simulink model "x64_32s_001_4ks"  (Simulink 7.4)
settings: Solver=ode45  StartTime=0.0  StopTime=100000  RelTol=1e-3
undefined symbols (not defined in the file): gcbh, ROACH, x64_32s_001_4ks_beam_mux_order_in_user_data_out, x64_32s_001_4ks_beam_mux_order_out_user_data_out, x64_32s_001_4ks_beam_mux_order_we_user_data_out, x64_32s_001_4ks_first_chan_user_data_out, x64_32s_001_4ks_gbe_dest_ip_user_data_out, x64_32s_001_4ks_gbe_dest_port_user_data_out, x64_32s_001_4ks_gbe_packet_cnt1_user_data_in, x64_32s_001_4ks_gbe_packet_cnt_user_data_in, x64_32s_001_4ks_heap_size_user_data_out, x64_32s_001_4ks_inst_seng_id0_user_data_in, x64_32s_001_4ks_last_chan_user_data_out, x64_32s_001_4ks_n_beams_user_data_out, x64_32s_001_4ks_qdr_in_cnt_user_data_in, x64_32s_001_4ks_status_user_data_in, x64_32s_001_4ks_x_window_mult_0_coeff_user_data_out, x64_32s_001_4ks_x_window_mult_1_coeff_user_data_out, x64_32s_001_4ks_x_window_mult_2_coeff_user_data_out, x64_32s_001_4ks_x_window_mult_3_coeff_user_data_out, x64_32s_001_4ks_xaui_cnt0_user_data_in, x64_32s_001_4ks_xaui_error_cnt0_user_data_in, x64_32s_001_4ks_xauibuf_out_cnt_user_data_in, x64_32s_001_4ks_xauibuf_window_cnt_user_data_in, x64_32s_001_4ks_y_window_mult_0_coeff_user_data_out, x64_32s_001_4ks_y_window_mult_1_coeff_user_data_out, x64_32s_001_4ks_y_window_mult_2_coeff_user_data_out, x64_32s_001_4ks_y_window_mult_3_coeff_user_data_out, x64_32s_001_4ks_y_window_mult_4_coeff_user_data_out, x64_32s_001_4ks_y_window_mult_5_coeff_user_data_out, x64_32s_001_4ks_y_window_mult_6_coeff_user_data_out, x64_32s_001_4ks_y_window_mult_7_coeff_user_data_out, xlBlockLoadCallback
libraries: xbsIndex_r4 x8559, casper_library_misc x308, casper_library_delays x33, casper_library_reorder x16, xps_library x5, ox_lib x1
(part 3 of 100)

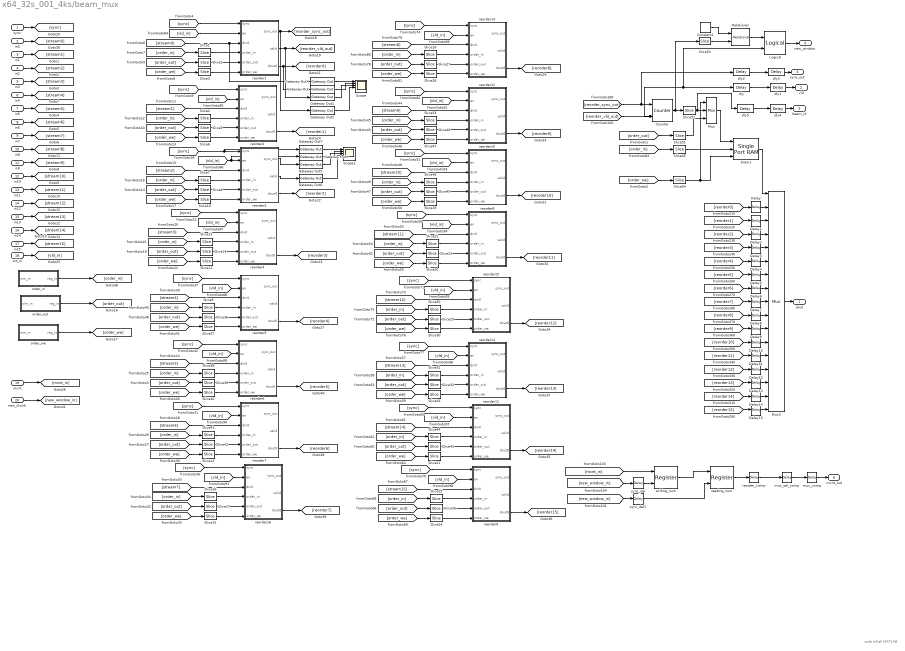
== system x64_32s_001_4ks/beam_mux ==
  note: sudo killall MATLAB
  sync : Inport
  in0 : Inport  Port=2
  in1 : Inport  Port=3
  in2 : Inport  Port=4
  in3 : Inport  Port=5
  in4 : Inport  Port=6
  in5 : Inport  Port=7
  in6 : Inport  Port=8
  in7 : Inport  Port=9
  in8 : Inport  Port=10
  in9 : Inport  Port=11
  in10 : Inport  Port=12
  in11 : Inport  Port=13
  in12 : Inport  Port=14
  in13 : Inport  Port=15
  in14 : Inport  Port=16
  in15 : Inport  Port=17
  vld_in : Inport  Port=18
  mcnt : Inport  Port=19
  new_chunk : Inport  Port=20
  Constant1 : xbsIndex_r4/Constant  arith_type=Unsigned  const=0  n_bits=n_int  bin_pt=0  explicit_period=on  period=1  equ=P=C  opselect=C  inp2=PCIN>>17  opr=+  inp1=P  carry=CIN  dbl_ovrd=off  block_type=constant
      settings: block_version=9.2.01
  Counter : xbsIndex_r4/Counter  cnt_type=Count Limited  cnt_to=(2^(n_int+n_mux))-1  operation=Up  start_count=0  cnt_by_val=1  arith_type=Unsigned  n_bits=n_int+n_mux  bin_pt=0  load_pin=off  rst=on  en=on  explicit_period=on  period=1  dbl_ovrd=off  use_behavioral_HDL=off  implementation=Fabric  block_type=counter
      settings: block_version=11.4
  Delay{,1..15} (16 alike) : xbsIndex_r4/Delay  en=off  latency=1  dbl_ovrd=off  reg_retiming=on  block_type=delay
      settings: xl_area=[0, 0, 0, 0, 0, 0, 0]  block_version=9.2.01
  Gateway Out{,1..7} (8 alike) : xbsIndex_r4/Gateway Out  hdl_port=off  timing_constraint=None  locs_specified=off  LOCs={}  block_type=gatewayout
      settings: infoedit="Gateway out block.  Converts Xilinx fixed point inputs into ouputs of type Simulink integer, double, or fixed point.<br><br>Hardware notes:  In hardware these blocks become top level output ports or are discarded, depending on how they are configured."  block_version=11.4
  Logical : xbsIndex_r4/Logical  logical_function=AND  inputs=2  en=off  latency=2  precision=Full  arith_type=Unsigned  n_bits=16  bin_pt=0  align_bp=on  dbl_ovrd=off  block_type=logical
      settings: block_version=11.4
  Mux : xbsIndex_r4/Mux  inputs=2  en=off  latency=0  precision=Full  arith_type="Signed  (2's comp)"  n_bits=8  bin_pt=2  quantization=Truncate  overflow=Wrap  dbl_ovrd=off  block_type=mux
      settings: xl_area=[0, 0, 0, 0, 0, 0, 0]  block_version=9.1.01
  Mux3 : xbsIndex_r4/Mux  inputs=16  en=off  latency=2  precision=Full  arith_type="Signed  (2's comp)"  n_bits=0  bin_pt=0  quantization=Truncate  overflow=Wrap  dbl_ovrd=off  block_type=mux
      settings: xl_area=[0, 0, 0, 0, 0, 0, 0]  block_version=9.2.01
  Relational : xbsIndex_r4/Relational  mode=a=b  en=off  latency=0  dbl_ovrd=off  block_type=relational
      settings: xl_area=[0, 0, 0, 0, 0, 0, 0]  block_version=9.2.01
  Scope : Scope  NumInputPorts=6
      settings: YMin=-5~-5~-5~-5~-5~-5  YMax=5~5~5~5~5~5
  Scope1 : Scope  NumInputPorts=6
      settings: YMin=-5~-5~-5~-5~-5~-5  YMax=5~5~5~5~5~5  SaveName=ScopeData7
  Slice{1..2,4..5,7..9,13..14,16..18,22..23,25..27,29,31..33,36,38..39,41..42,44..46,48..51} (33 alike) : xbsIndex_r4/Slice  nbits=n_mux  boolean_output=off  mode=Lower Bit Location + Width  bit1=0  base1=MSB of Input  bit0=0  base0=LSB of Input  dbl_ovrd=off  block_type=slice
  Slice10 : xbsIndex_r4/Slice  nbits=1  boolean_output=on  mode=Lower Bit Location + Width  bit1=0  base1=MSB of Input  bit0=2  base0=LSB of Input  dbl_ovrd=off  block_type=slice
  Slice11 : xbsIndex_r4/Slice  nbits=1  boolean_output=on  mode=Lower Bit Location + Width  bit1=0  base1=MSB of Input  bit0=3  base0=LSB of Input  dbl_ovrd=off  block_type=slice
  Slice12 : xbsIndex_r4/Slice  nbits=1  boolean_output=on  mode=Lower Bit Location + Width  bit1=0  base1=MSB of Input  bit0=6  base0=LSB of Input  dbl_ovrd=off  block_type=slice
  Slice15 : xbsIndex_r4/Slice  nbits=1  boolean_output=on  mode=Lower Bit Location + Width  bit1=0  base1=MSB of Input  bit0=7  base0=LSB of Input  dbl_ovrd=off  block_type=slice
  Slice19 : xbsIndex_r4/Slice  nbits=1  boolean_output=on  mode=Lower Bit Location + Width  bit1=0  base1=MSB of Input  bit0=10  base0=LSB of Input  dbl_ovrd=off  block_type=slice
  Slice20 : xbsIndex_r4/Slice  nbits=1  boolean_output=on  mode=Lower Bit Location + Width  bit1=0  base1=MSB of Input  bit0=11  base0=LSB of Input  dbl_ovrd=off  block_type=slice
  Slice21 : xbsIndex_r4/Slice  nbits=1  boolean_output=on  mode=Lower Bit Location + Width  bit1=0  base1=MSB of Input  bit0=14  base0=LSB of Input  dbl_ovrd=off  block_type=slice
  Slice24 : xbsIndex_r4/Slice  nbits=1  boolean_output=on  mode=Lower Bit Location + Width  bit1=0  base1=MSB of Input  bit0=15  base0=LSB of Input  dbl_ovrd=off  block_type=slice
  Slice28 : xbsIndex_r4/Slice  nbits=1  boolean_output=on  mode=Lower Bit Location + Width  bit1=0  base1=MSB of Input  bit0=8  base0=LSB of Input  dbl_ovrd=off  block_type=slice
  Slice3 : xbsIndex_r4/Slice  nbits=1  boolean_output=on  mode=Lower Bit Location + Width  bit1=0  base1=MSB of Input  bit0=0  base0=LSB of Input  dbl_ovrd=off  block_type=slice
  Slice30 : xbsIndex_r4/Slice  nbits=1  boolean_output=on  mode=Lower Bit Location + Width  bit1=0  base1=MSB of Input  bit0=12  base0=LSB of Input  dbl_ovrd=off  block_type=slice
  Slice34 : xbsIndex_r4/Slice  nbits=1  boolean_output=on  mode=Upper Bit Location + Width  bit1=0  base1=MSB of Input  bit0=11  base0=LSB of Input  dbl_ovrd=off  block_type=slice
  Slice35 : xbsIndex_r4/Slice  nbits=log2(16)  boolean_output=off  mode=Lower Bit Location + Width  bit1=0  base1=MSB of Input  bit0=0  base0=LSB of Input  dbl_ovrd=off  block_type=slice
  Slice37 : xbsIndex_r4/Slice  nbits=1  boolean_output=on  mode=Lower Bit Location + Width  bit1=0  base1=MSB of Input  bit0=4  base0=LSB of Input  dbl_ovrd=off  block_type=slice
  Slice40 : xbsIndex_r4/Slice  nbits=1  boolean_output=on  mode=Lower Bit Location + Width  bit1=0  base1=MSB of Input  bit0=5  base0=LSB of Input  dbl_ovrd=off  block_type=slice
  Slice43 : xbsIndex_r4/Slice  nbits=1  boolean_output=on  mode=Lower Bit Location + Width  bit1=0  base1=MSB of Input  bit0=13  base0=LSB of Input  dbl_ovrd=off  block_type=slice
  Slice47 : xbsIndex_r4/Slice  nbits=1  boolean_output=on  mode=Lower Bit Location + Width  bit1=0  base1=MSB of Input  bit0=9  base0=LSB of Input  dbl_ovrd=off  block_type=slice
  Slice52 : xbsIndex_r4/Slice  nbits=n_mux  boolean_output=off  mode=Upper Bit Location + Width  bit1=0  base1=MSB of Input  bit0=0  base0=LSB of Input  dbl_ovrd=off  block_type=slice
  Slice53 : xbsIndex_r4/Slice  nbits=n_int  boolean_output=off  mode=Lower Bit Location + Width  bit1=0  base1=MSB of Input  bit0=0  base0=LSB of Input  dbl_ovrd=off  block_type=slice
  Slice6 : xbsIndex_r4/Slice  nbits=1  boolean_output=on  mode=Lower Bit Location + Width  bit1=0  base1=MSB of Input  bit0=1  base0=LSB of Input  dbl_ovrd=off  block_type=slice
  bram1 : xbsIndex_r4/Single Port RAM  depth=2^n_mux  initVector=[0 0 0 0 1 1 1 1 0 0 0 0 1 1 1 1]  distributed_mem=Distributed memory  write_mode=Read Before Write  rst=off  init_reg=0  en=off  latency=1  dbl_ovrd=off  optimize=Area  use_rpm=off  block_type=spram
      settings: xl_area=[0, 0, 0, 0, 0, 0, 0]  block_version=9.1.01
  dly : xbsIndex_r4/Delay  en=off  latency=1  dbl_ovrd=off  reg_retiming=off  block_type=delay
      settings: block_version=11.4
  dly1 : xbsIndex_r4/Delay  en=off  latency=2  dbl_ovrd=off  reg_retiming=off  block_type=delay
      settings: block_version=11.4
  dly2 : xbsIndex_r4/Delay  en=off  latency=1  dbl_ovrd=off  reg_retiming=off  block_type=delay
      settings: block_version=11.4
  dly3 : xbsIndex_r4/Delay  en=off  latency=2  dbl_ovrd=off  reg_retiming=off  block_type=delay
      settings: block_version=11.4
  dly4 : xbsIndex_r4/Delay  en=off  latency=2  dbl_ovrd=off  reg_retiming=off  block_type=delay
      settings: block_version=11.4
  dly5 : xbsIndex_r4/Delay  en=off  latency=1  dbl_ovrd=off  reg_retiming=off  block_type=delay
      settings: block_version=11.4
  mux_comp : xbsIndex_r4/Delay  en=off  latency=2  dbl_ovrd=off  reg_retiming=on  block_type=delay
      settings: xl_area=[0, 0, 0, 0, 0, 0, 0]  block_version=9.2.01
  mux_sel_comp : xbsIndex_r4/Delay  en=off  latency=1  dbl_ovrd=off  reg_retiming=on  block_type=delay
      settings: xl_area=[0, 0, 0, 0, 0, 0, 0]  block_version=9.2.01
  order_in : Subsystem  Tag=xps:sw_reg  mask{io_dir=From Processor, arith_type=Unsigned, bitwidth=32, bin_pt=0, sample_period=1, gw_name=x64_32s_001_4ks_beam_mux_order_in_user_data_out}
      mask prompts: io_dir "I/O direction"; arith_type "Data Type"; bitwidth "Data bitwidth"; bin_pt "Data binary point"; sample_period "Sample period"; gw_name "Mask Parameter 6:"
      mask init:
      | register_mask;
      mask StyleString: popup(From Processor|To Processor),popup(Unsigned|Signed  (2's comp)),edit,edit,edit,edit
  order_out : Subsystem  Tag=xps:sw_reg  mask{io_dir=From Processor, arith_type=Unsigned, bitwidth=32, bin_pt=0, sample_period=1, gw_name=x64_32s_001_4ks_beam_mux_order_out_user_data_out}
      mask prompts: io_dir "I/O direction"; arith_type "Data Type"; bitwidth "Data bitwidth"; bin_pt "Data binary point"; sample_period "Sample period"; gw_name "Mask Parameter 6:"
      mask init:
      | register_mask;
      mask StyleString: popup(From Processor|To Processor),popup(Unsigned|Signed  (2's comp)),edit,edit,edit,edit
  order_we : Subsystem  Tag=xps:sw_reg  mask{io_dir=From Processor, arith_type=Unsigned, bitwidth=32, bin_pt=0, sample_period=1, gw_name=x64_32s_001_4ks_beam_mux_order_we_user_data_out}
      mask prompts: io_dir "I/O direction"; arith_type "Data Type"; bitwidth "Data bitwidth"; bin_pt "Data binary point"; sample_period "Sample period"; gw_name "Mask Parameter 6:"
      mask init:
      | register_mask;
      mask StyleString: popup(From Processor|To Processor),popup(Unsigned|Signed  (2's comp)),edit,edit,edit,edit
  reading_num : xbsIndex_r4/Register  init=0  rst=off  en=on  dbl_ovrd=off  block_type=register
      settings: block_version=11.4
  reorder1 : Subsystem  AncestorBlock=casper_library_reorder/reorder  mask(reorder){map=getfield( getfield( get_param( gcb,'UserData' ), 'parameters'),'map'), n_inputs=1, bram_latency=1, map_latency=1, double_buffer=1, bram_map=off}
      mask prompts: map "Output Order:"; n_inputs "Number of inputs"; bram_latency "BRAM Latency"; map_latency "Map Latency"; double_buffer "Double Buffer"; bram_map "Use BlockRAM for Map"
      settings: AttributesFormatString=order=2
      mask Help: eval('xlWeb(''http://casper.berkeley.edu/wiki/Reorder'')')
  reorder10 : Subsystem  AncestorBlock=casper_library_reorder/reorder  mask(reorder){map=getfield( getfield( get_param( gcb,'UserData' ), 'parameters'),'map'), n_inputs=1, bram_latency=1, map_latency=1, double_buffer=1, bram_map=off}
      mask prompts: map "Output Order:"; n_inputs "Number of inputs"; bram_latency "BRAM Latency"; map_latency "Map Latency"; double_buffer "Double Buffer"; bram_map "Use BlockRAM for Map"
      settings: AttributesFormatString=order=2
      mask Help: eval('xlWeb(''http://casper.berkeley.edu/wiki/Reorder'')')
  reorder11 : Subsystem  AncestorBlock=casper_library_reorder/reorder  mask(reorder){map=getfield( getfield( get_param( gcb,'UserData' ), 'parameters'),'map'), n_inputs=1, bram_latency=1, map_latency=1, double_buffer=1, bram_map=off}
      mask prompts: map "Output Order:"; n_inputs "Number of inputs"; bram_latency "BRAM Latency"; map_latency "Map Latency"; double_buffer "Double Buffer"; bram_map "Use BlockRAM for Map"
      settings: AttributesFormatString=order=2
      mask Help: eval('xlWeb(''http://casper.berkeley.edu/wiki/Reorder'')')
  reorder12 : Subsystem  AncestorBlock=casper_library_reorder/reorder  mask(reorder){map=getfield( getfield( get_param( gcb,'UserData' ), 'parameters'),'map'), n_inputs=1, bram_latency=1, map_latency=1, double_buffer=1, bram_map=off}
      mask prompts: map "Output Order:"; n_inputs "Number of inputs"; bram_latency "BRAM Latency"; map_latency "Map Latency"; double_buffer "Double Buffer"; bram_map "Use BlockRAM for Map"
      settings: AttributesFormatString=order=2
      mask Help: eval('xlWeb(''http://casper.berkeley.edu/wiki/Reorder'')')
  reorder13 : Subsystem  AncestorBlock=casper_library_reorder/reorder  mask(reorder){map=getfield( getfield( get_param( gcb,'UserData' ), 'parameters'),'map'), n_inputs=1, bram_latency=1, map_latency=1, double_buffer=1, bram_map=off}
      mask prompts: map "Output Order:"; n_inputs "Number of inputs"; bram_latency "BRAM Latency"; map_latency "Map Latency"; double_buffer "Double Buffer"; bram_map "Use BlockRAM for Map"
      settings: AttributesFormatString=order=2
      mask Help: eval('xlWeb(''http://casper.berkeley.edu/wiki/Reorder'')')
  reorder14 : Subsystem  AncestorBlock=casper_library_reorder/reorder  mask(reorder){map=getfield( getfield( get_param( gcb,'UserData' ), 'parameters'),'map'), n_inputs=1, bram_latency=1, map_latency=1, double_buffer=1, bram_map=off}
      mask prompts: map "Output Order:"; n_inputs "Number of inputs"; bram_latency "BRAM Latency"; map_latency "Map Latency"; double_buffer "Double Buffer"; bram_map "Use BlockRAM for Map"
      settings: AttributesFormatString=order=2
      mask Help: eval('xlWeb(''http://casper.berkeley.edu/wiki/Reorder'')')
  reorder15 : Subsystem  AncestorBlock=casper_library_reorder/reorder  mask(reorder){map=getfield( getfield( get_param( gcb,'UserData' ), 'parameters'),'map'), n_inputs=1, bram_latency=1, map_latency=1, double_buffer=1, bram_map=off}
      mask prompts: map "Output Order:"; n_inputs "Number of inputs"; bram_latency "BRAM Latency"; map_latency "Map Latency"; double_buffer "Double Buffer"; bram_map "Use BlockRAM for Map"
      settings: AttributesFormatString=order=2
      mask Help: eval('xlWeb(''http://casper.berkeley.edu/wiki/Reorder'')')
  reorder16 : Subsystem  AncestorBlock=casper_library_reorder/reorder  mask(reorder){map=getfield( getfield( get_param( gcb,'UserData' ), 'parameters'),'map'), n_inputs=1, bram_latency=1, map_latency=1, double_buffer=1, bram_map=off}
      mask prompts: map "Output Order:"; n_inputs "Number of inputs"; bram_latency "BRAM Latency"; map_latency "Map Latency"; double_buffer "Double Buffer"; bram_map "Use BlockRAM for Map"
      settings: AttributesFormatString=order=2
      mask Help: eval('xlWeb(''http://casper.berkeley.edu/wiki/Reorder'')')
  reorder2 : Subsystem  AncestorBlock=casper_library_reorder/reorder  mask(reorder){map=getfield( getfield( get_param( gcb,'UserData' ), 'parameters'),'map'), n_inputs=1, bram_latency=1, map_latency=1, double_buffer=1, bram_map=off}
      mask prompts: map "Output Order:"; n_inputs "Number of inputs"; bram_latency "BRAM Latency"; map_latency "Map Latency"; double_buffer "Double Buffer"; bram_map "Use BlockRAM for Map"
      settings: AttributesFormatString=order=2
      mask Help: eval('xlWeb(''http://casper.berkeley.edu/wiki/Reorder'')')
  reorder3 : Subsystem  AncestorBlock=casper_library_reorder/reorder  mask(reorder){map=getfield( getfield( get_param( gcb,'UserData' ), 'parameters'),'map'), n_inputs=1, bram_latency=1, map_latency=1, double_buffer=1, bram_map=off}
      mask prompts: map "Output Order:"; n_inputs "Number of inputs"; bram_latency "BRAM Latency"; map_latency "Map Latency"; double_buffer "Double Buffer"; bram_map "Use BlockRAM for Map"
      settings: AttributesFormatString=order=2
      mask Help: eval('xlWeb(''http://casper.berkeley.edu/wiki/Reorder'')')
  reorder4 : Subsystem  AncestorBlock=casper_library_reorder/reorder  mask(reorder){map=getfield( getfield( get_param( gcb,'UserData' ), 'parameters'),'map'), n_inputs=1, bram_latency=1, map_latency=1, double_buffer=1, bram_map=off}
      mask prompts: map "Output Order:"; n_inputs "Number of inputs"; bram_latency "BRAM Latency"; map_latency "Map Latency"; double_buffer "Double Buffer"; bram_map "Use BlockRAM for Map"
      settings: AttributesFormatString=order=2
      mask Help: eval('xlWeb(''http://casper.berkeley.edu/wiki/Reorder'')')
  reorder5 : Subsystem  AncestorBlock=casper_library_reorder/reorder  mask(reorder){map=getfield( getfield( get_param( gcb,'UserData' ), 'parameters'),'map'), n_inputs=1, bram_latency=1, map_latency=1, double_buffer=1, bram_map=off}
      mask prompts: map "Output Order:"; n_inputs "Number of inputs"; bram_latency "BRAM Latency"; map_latency "Map Latency"; double_buffer "Double Buffer"; bram_map "Use BlockRAM for Map"
      settings: AttributesFormatString=order=2
      mask Help: eval('xlWeb(''http://casper.berkeley.edu/wiki/Reorder'')')
  reorder6 : Subsystem  AncestorBlock=casper_library_reorder/reorder  mask(reorder){map=getfield( getfield( get_param( gcb,'UserData' ), 'parameters'),'map'), n_inputs=1, bram_latency=1, map_latency=1, double_buffer=1, bram_map=off}
      mask prompts: map "Output Order:"; n_inputs "Number of inputs"; bram_latency "BRAM Latency"; map_latency "Map Latency"; double_buffer "Double Buffer"; bram_map "Use BlockRAM for Map"
      settings: AttributesFormatString=order=2
      mask Help: eval('xlWeb(''http://casper.berkeley.edu/wiki/Reorder'')')
  reorder7 : Subsystem  AncestorBlock=casper_library_reorder/reorder  mask(reorder){map=getfield( getfield( get_param( gcb,'UserData' ), 'parameters'),'map'), n_inputs=1, bram_latency=1, map_latency=1, double_buffer=1, bram_map=off}
      mask prompts: map "Output Order:"; n_inputs "Number of inputs"; bram_latency "BRAM Latency"; map_latency "Map Latency"; double_buffer "Double Buffer"; bram_map "Use BlockRAM for Map"
      settings: AttributesFormatString=order=2
      mask Help: eval('xlWeb(''http://casper.berkeley.edu/wiki/Reorder'')')
  reorder8 : Subsystem  AncestorBlock=casper_library_reorder/reorder  mask(reorder){map=getfield( getfield( get_param( gcb,'UserData' ), 'parameters'),'map'), n_inputs=1, bram_latency=1, map_latency=1, double_buffer=1, bram_map=off}
      mask prompts: map "Output Order:"; n_inputs "Number of inputs"; bram_latency "BRAM Latency"; map_latency "Map Latency"; double_buffer "Double Buffer"; bram_map "Use BlockRAM for Map"
      settings: AttributesFormatString=order=2
      mask Help: eval('xlWeb(''http://casper.berkeley.edu/wiki/Reorder'')')
  reorder9 : Subsystem  AncestorBlock=casper_library_reorder/reorder  mask(reorder){map=getfield( getfield( get_param( gcb,'UserData' ), 'parameters'),'map'), n_inputs=1, bram_latency=1, map_latency=1, double_buffer=1, bram_map=off}
      mask prompts: map "Output Order:"; n_inputs "Number of inputs"; bram_latency "BRAM Latency"; map_latency "Map Latency"; double_buffer "Double Buffer"; bram_map "Use BlockRAM for Map"
      settings: AttributesFormatString=order=2
      mask Help: eval('xlWeb(''http://casper.berkeley.edu/wiki/Reorder'')')
  reorder_comp : xbsIndex_r4/Delay  en=off  latency=3  dbl_ovrd=off  reg_retiming=on  block_type=delay
      settings: xl_area=[0, 0, 0, 0, 0, 0, 0]  block_version=9.2.01
  sync_del : xbsIndex_r4/Delay  en=off  latency=1  dbl_ovrd=off  reg_retiming=on  block_type=delay
      settings: xl_area=[0, 0, 0, 0, 0, 0, 0]  block_version=9.2.01
  sync_del1 : xbsIndex_r4/Delay  en=off  latency=1  dbl_ovrd=off  reg_retiming=on  block_type=delay
      settings: xl_area=[0, 0, 0, 0, 0, 0, 0]  block_version=9.2.01
  writing_num : xbsIndex_r4/Register  init=0  rst=off  en=on  dbl_ovrd=off  block_type=register
      settings: block_version=11.4
  dout : Outport
  vld : Outport  Port=2
  sync_out : Outport  Port=3
  new_window : Outport  Port=4
  beam_id : Outport  Port=5
  mcnt_out : Outport  Port=6
  wires:
  in0/1 -> [stream0]
  in1/1 -> [stream1]
  in2/1 -> [stream2]
  in3/1 -> [stream3]
  in4/1 -> [stream4]
  in5/1 -> [stream5]
  in6/1 -> [stream6]
  in7/1 -> [stream7]
  in8/1 -> [stream8]
  in9/1 -> [stream9]
  in10/1 -> [stream10]
  in11/1 -> [stream11]
  in12/1 -> [stream12]
  in13/1 -> [stream13]
  in14/1 -> [stream14]
  in15/1 -> [stream15]
  [reorder0] -> Delay/1
  [reorder1] -> Delay1/1
  [reorder2] -> Delay2/1
  [reorder3] -> Delay3/1
  [reorder4] -> Delay4/1
  [reorder5] -> Delay5/1
  [reorder6] -> Delay6/1
  [reorder7] -> Delay7/1
  [reorder8] -> Delay8/1
  [reorder9] -> Delay9/1
  [reorder10] -> Delay10/1
  [reorder11] -> Delay11/1
  [reorder12] -> Delay12/1
  [reorder13] -> Delay13/1
  [reorder14] -> Delay14/1
  [reorder15] -> Delay15/1
  Mux3/1 -> dout/1
  sync/1 -> [sync]
  order_out/1(reg_in) -> [order_out]
  order_we/1(reg_in) -> [order_we]
  reorder1/1(sync_out) -> [reorder_sync_out], Gateway Out2/1
  reorder1/2(valid) -> [reorder_vld_out], Gateway Out1/1
  [reorder_sync_out] -> Counter/1, dly2/1
  [reorder_vld_out] -> Counter/2, dly/1, Logical/2
  [order_we] -> Slice34/1
  Slice34/1 -> bram1/3, Mux/1
  Slice35/1 -> bram1/2
  order_in/1(reg_in) -> [order_in]
  [sync] -> reorder1/1(sync)
  [vld_in] -> reorder1/2(en)
  [stream0] -> Gateway Out4/1, reorder1/3(din0)
  [order_in] -> Slice1/1
  [order_we] -> Slice3/1
  Slice1/1 -> reorder1/4(order_in)
  Slice3/1 -> reorder1/6(order_we)
  reorder1/3(dout0) -> [reorder0], Gateway Out/1
  [order_out] -> Slice2/1
  Slice2/1 -> reorder1/5(order_out)
  [sync] -> reorder2/1(sync)
  [vld_in] -> reorder2/2(en)
  [stream1] -> reorder2/3(din0)
  [order_in] -> Slice4/1
  [order_we] -> Slice6/1
  Slice4/1 -> reorder2/4(order_in)
  Slice6/1 -> reorder2/6(order_we)
  reorder2/3(dout0) -> [reorder1]
  [order_out] -> Slice5/1
  Slice5/1 -> reorder2/5(order_out)
  [sync] -> Gateway Out7/1, reorder3/1(sync)
  [vld_in] -> Gateway Out3/1, reorder3/2(en)
  [stream2] -> reorder3/3(din0)
  [order_in] -> Slice7/1
  [order_we] -> Slice10/1
  Slice7/1 -> reorder3/4(order_in)
  Slice10/1 -> reorder3/6(order_we)
  reorder3/3(dout0) -> [reorder2]
  [order_out] -> Slice8/1
  Slice8/1 -> reorder3/5(order_out)
  [sync] -> reorder4/1(sync)
  [vld_in] -> reorder4/2(en)
  [stream3] -> reorder4/3(din0)
  [order_in] -> Slice13/1
  [order_we] -> Slice11/1
  Slice13/1 -> reorder4/4(order_in)
  Slice11/1 -> reorder4/6(order_we)
  reorder4/3(dout0) -> [reorder3]
  [order_out] -> Slice14/1
  Slice14/1 -> reorder4/5(order_out)
  [sync] -> reorder5/1(sync)
  [vld_in] -> reorder5/2(en)
  [stream4] -> reorder5/3(din0)
  [order_in] -> Slice9/1
  [order_we] -> Slice37/1
  Slice9/1 -> reorder5/4(order_in)
  Slice37/1 -> reorder5/6(order_we)
  reorder5/3(dout0) -> [reorder4]
  [order_out] -> Slice36/1
  Slice36/1 -> reorder5/5(order_out)
  [sync] -> reorder6/1(sync)
  [vld_in] -> reorder6/2(en)
  [stream5] -> reorder6/3(din0)
  [order_in] -> Slice38/1
  [order_we] -> Slice40/1
  Slice38/1 -> reorder6/4(order_in)
  Slice40/1 -> reorder6/6(order_we)
  reorder6/3(dout0) -> [reorder5]
  [order_out] -> Slice39/1
  Slice39/1 -> reorder6/5(order_out)
  [sync] -> reorder7/1(sync)
  [vld_in] -> reorder7/2(en)
  [stream6] -> reorder7/3(din0)
  [order_in] -> Slice41/1
  [order_we] -> Slice12/1
  Slice41/1 -> reorder7/4(order_in)
  Slice12/1 -> reorder7/6(order_we)
  reorder7/3(dout0) -> [reorder6]
  [order_out] -> Slice42/1
  Slice42/1 -> reorder7/5(order_out)
  [sync] -> reorder16/1(sync)
  [vld_in] -> reorder16/2(en)
  [stream7] -> reorder16/3(din0)
  [order_in] -> Slice16/1
  [order_we] -> Slice15/1
  Slice16/1 -> reorder16/4(order_in)
  Slice15/1 -> reorder16/6(order_we)
  reorder16/3(dout0) -> [reorder7]
  [order_out] -> Slice17/1
  Slice17/1 -> reorder16/5(order_out)
  [sync] -> reorder13/1(sync)
  [vld_in] -> reorder13/2(en)
  [stream8] -> reorder13/3(din0)
  [order_in] -> Slice18/1
  [order_we] -> Slice28/1
  Slice18/1 -> reorder13/4(order_in)
  Slice28/1 -> reorder13/6(order_we)
  reorder13/3(dout0) -> [reorder8]
  [order_out] -> Slice27/1
  Slice27/1 -> reorder13/5(order_out)
  [sync] -> reorder12/1(sync)
  [vld_in] -> reorder12/2(en)
  [stream9] -> reorder12/3(din0)
  [order_in] -> Slice33/1
  [order_we] -> Slice47/1
  Slice33/1 -> reorder12/4(order_in)
  Slice47/1 -> reorder12/6(order_we)
  reorder12/3(dout0) -> [reorder9]
  [order_out] -> Slice46/1
  Slice46/1 -> reorder12/5(order_out)
  [sync] -> reorder10/1(sync)
  [vld_in] -> reorder10/2(en)
  [stream10] -> reorder10/3(din0)
  [order_in] -> Slice48/1
  [order_we] -> Slice19/1
  Slice48/1 -> reorder10/4(order_in)
  Slice19/1 -> reorder10/6(order_we)
  reorder10/3(dout0) -> [reorder10]
  [order_out] -> Slice49/1
  Slice49/1 -> reorder10/5(order_out)
  [sync] -> reorder8/1(sync)
  [vld_in] -> reorder8/2(en)
  [stream11] -> reorder8/3(din0)
  [order_in] -> Slice22/1
  [order_we] -> Slice20/1
  Slice22/1 -> reorder8/4(order_in)
  Slice20/1 -> reorder8/6(order_we)
  reorder8/3(dout0) -> [reorder11]
  [order_out] -> Slice23/1
  Slice23/1 -> reorder8/5(order_out)
  [sync] -> reorder15/1(sync)
  [vld_in] -> reorder15/2(en)
  [stream12] -> reorder15/3(din0)
  [order_in] -> Slice50/1
  [order_we] -> Slice30/1
  Slice50/1 -> reorder15/4(order_in)
  Slice30/1 -> reorder15/6(order_we)
  reorder15/3(dout0) -> [reorder12]
  [order_out] -> Slice29/1
  Slice29/1 -> reorder15/5(order_out)
  [sync] -> reorder14/1(sync)
  [vld_in] -> reorder14/2(en)
  [stream13] -> reorder14/3(din0)
  [order_in] -> Slice31/1
  [order_we] -> Slice43/1
  Slice31/1 -> reorder14/4(order_in)
  Slice43/1 -> reorder14/6(order_we)
  reorder14/3(dout0) -> [reorder13]
  [order_out] -> Slice32/1
  Slice32/1 -> reorder14/5(order_out)
  [sync] -> reorder11/1(sync)
  [vld_in] -> reorder11/2(en)
  [stream14] -> reorder11/3(din0)
  [order_in] -> Slice44/1
  [order_we] -> Slice21/1
  Slice44/1 -> reorder11/4(order_in)
  Slice21/1 -> reorder11/6(order_we)
  reorder11/3(dout0) -> [reorder14]
  [order_out] -> Slice45/1
  Slice45/1 -> reorder11/5(order_out)
  [sync] -> reorder9/1(sync)
  [vld_in] -> reorder9/2(en)
  [stream15] -> reorder9/3(din0)
  [order_in] -> Slice25/1
  [order_we] -> Slice24/1
  Slice25/1 -> reorder9/4(order_in)
  Slice24/1 -> reorder9/6(order_we)
  reorder9/3(dout0) -> [reorder15]
  [order_out] -> Slice26/1
  Slice26/1 -> reorder9/5(order_out)
  Counter/1 -> Slice52/1, Slice53/1
  Slice51/1 -> Mux/3
  Mux/1 -> bram1/1
  dly/1 -> dly1/1
  dly1/1 -> vld/1
  Slice52/1 -> Mux/2, dly5/1
  dly2/1 -> dly3/1
  dly3/1 -> sync_out/1
  vld_in/1 -> [vld_in]
  Gateway Out4/1 -> Scope/1
  Gateway Out/1 -> Scope/2
  Gateway Out1/1 -> Scope/3
  Delay/1 -> Mux3/2
  Delay1/1 -> Mux3/3
  Delay2/1 -> Mux3/4
  Delay3/1 -> Mux3/5
  Delay4/1 -> Mux3/6
  Delay5/1 -> Mux3/7
  Delay6/1 -> Mux3/8
  Delay7/1 -> Mux3/9
  Delay8/1 -> Mux3/10
  Delay9/1 -> Mux3/11
  Delay10/1 -> Mux3/12
  Delay11/1 -> Mux3/13
  Delay12/1 -> Mux3/14
  Delay13/1 -> Mux3/15
  Delay14/1 -> Mux3/16
  Delay15/1 -> Mux3/17
  Constant1/1 -> Relational/1
  Relational/1 -> Logical/1
  Logical/1 -> new_window/1
  Slice53/1 -> Relational/2
  dly4/1 -> beam_id/1
  bram1/1 -> Mux3/1
  dly5/1 -> dly4/1
  writing_num/1 -> reading_num/1
  mcnt/1 -> [mcnt_in]
  [mcnt_in] -> writing_num/1
  new_chunk/1 -> [new_window_in]
  [new_window_in] -> sync_del/1
  [new_window_in] -> sync_del1/1
  reading_num/1 -> reorder_comp/1
  reorder_comp/1 -> mux_sel_comp/1
  mux_sel_comp/1 -> mux_comp/1
  mux_comp/1 -> mcnt_out/1
  sync_del/1 -> writing_num/2
  sync_del1/1 -> reading_num/2
  Gateway Out2/1 -> Scope/4
  Gateway Out7/1 -> Scope1/1
  Gateway Out3/1 -> Scope1/2
  Gateway Out5/1 -> Scope1/3
  Gateway Out6/1 -> Scope1/4
  reorder3/1(sync_out) -> Gateway Out5/1
  reorder3/2(valid) -> Gateway Out6/1
  [order_out] -> Slice51/1
  [order_in] -> Slice35/1

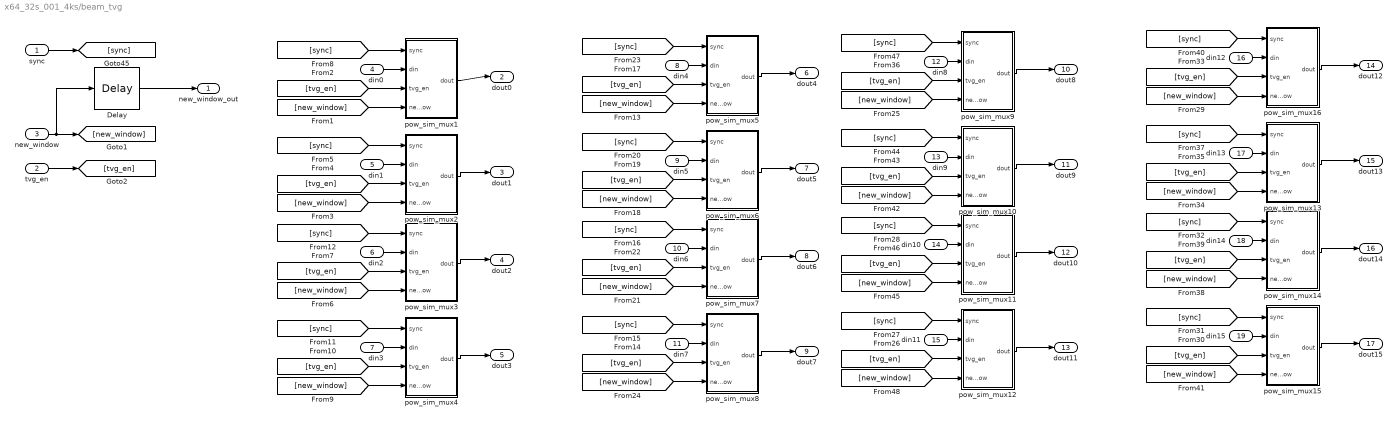
== system x64_32s_001_4ks/beam_tvg ==
  sync : Inport
  tvg_en : Inport  Port=2
  new_window : Inport  Port=3
  din0 : Inport  Port=4
  din1 : Inport  Port=5
  din2 : Inport  Port=6
  din3 : Inport  Port=7
  din4 : Inport  Port=8
  din5 : Inport  Port=9
  din6 : Inport  Port=10
  din7 : Inport  Port=11
  din8 : Inport  Port=12
  din9 : Inport  Port=13
  din10 : Inport  Port=14
  din11 : Inport  Port=15
  din12 : Inport  Port=16
  din13 : Inport  Port=17
  din14 : Inport  Port=18
  din15 : Inport  Port=19
  Delay : xbsIndex_r4/Delay  en=off  latency=1  dbl_ovrd=off  reg_retiming=off  block_type=delay
      settings: block_version=11.4
  pow_sim_mux1 : Subsystem  mask{valid_block_bits=7+3, beam_block_bits=7, y_beam_val=0, ant_bits=3}
      mask prompts: valid_block_bits "Size of valid block (2^?)"; beam_block_bits "beam block size (2^?)"; y_beam_val "Y-beam value"; ant_bits "Number of antennas (2^?)"
  pow_sim_mux10 : Subsystem  mask{valid_block_bits=7+3, beam_block_bits=7, y_beam_val=9, ant_bits=3}
      mask prompts: valid_block_bits "Size of valid block (2^?)"; beam_block_bits "beam block size (2^?)"; y_beam_val "Y-beam value"; ant_bits "Number of antennas (2^?)"
  pow_sim_mux11 : Subsystem  mask{valid_block_bits=7+3, beam_block_bits=7, y_beam_val=10, ant_bits=3}
      mask prompts: valid_block_bits "Size of valid block (2^?)"; beam_block_bits "beam block size (2^?)"; y_beam_val "Y-beam value"; ant_bits "Number of antennas (2^?)"
  pow_sim_mux12 : Subsystem  mask{valid_block_bits=7+3, beam_block_bits=7, y_beam_val=11, ant_bits=3}
      mask prompts: valid_block_bits "Size of valid block (2^?)"; beam_block_bits "beam block size (2^?)"; y_beam_val "Y-beam value"; ant_bits "Number of antennas (2^?)"
  pow_sim_mux13 : Subsystem  mask{valid_block_bits=7+3, beam_block_bits=7, y_beam_val=13, ant_bits=3}
      mask prompts: valid_block_bits "Size of valid block (2^?)"; beam_block_bits "beam block size (2^?)"; y_beam_val "Y-beam value"; ant_bits "Number of antennas (2^?)"
  pow_sim_mux14 : Subsystem  mask{valid_block_bits=7+3, beam_block_bits=7, y_beam_val=14, ant_bits=3}
      mask prompts: valid_block_bits "Size of valid block (2^?)"; beam_block_bits "beam block size (2^?)"; y_beam_val "Y-beam value"; ant_bits "Number of antennas (2^?)"
  pow_sim_mux15 : Subsystem  mask{valid_block_bits=7+3, beam_block_bits=7, y_beam_val=15, ant_bits=3}
      mask prompts: valid_block_bits "Size of valid block (2^?)"; beam_block_bits "beam block size (2^?)"; y_beam_val "Y-beam value"; ant_bits "Number of antennas (2^?)"
  pow_sim_mux16 : Subsystem  mask{valid_block_bits=7+3, beam_block_bits=7, y_beam_val=12, ant_bits=3}
      mask prompts: valid_block_bits "Size of valid block (2^?)"; beam_block_bits "beam block size (2^?)"; y_beam_val "Y-beam value"; ant_bits "Number of antennas (2^?)"
  pow_sim_mux2 : Subsystem  mask{valid_block_bits=7+3, beam_block_bits=7, y_beam_val=1, ant_bits=3}
      mask prompts: valid_block_bits "Size of valid block (2^?)"; beam_block_bits "beam block size (2^?)"; y_beam_val "Y-beam value"; ant_bits "Number of antennas (2^?)"
  pow_sim_mux3 : Subsystem  mask{valid_block_bits=7+3, beam_block_bits=7, y_beam_val=2, ant_bits=3}
      mask prompts: valid_block_bits "Size of valid block (2^?)"; beam_block_bits "beam block size (2^?)"; y_beam_val "Y-beam value"; ant_bits "Number of antennas (2^?)"
  pow_sim_mux4 : Subsystem  mask{valid_block_bits=7+3, beam_block_bits=7, y_beam_val=3, ant_bits=3}
      mask prompts: valid_block_bits "Size of valid block (2^?)"; beam_block_bits "beam block size (2^?)"; y_beam_val "Y-beam value"; ant_bits "Number of antennas (2^?)"
  pow_sim_mux5 : Subsystem  mask{valid_block_bits=7+3, beam_block_bits=7, y_beam_val=4, ant_bits=3}
      mask prompts: valid_block_bits "Size of valid block (2^?)"; beam_block_bits "beam block size (2^?)"; y_beam_val "Y-beam value"; ant_bits "Number of antennas (2^?)"
  pow_sim_mux6 : Subsystem  mask{valid_block_bits=7+3, beam_block_bits=7, y_beam_val=5, ant_bits=3}
      mask prompts: valid_block_bits "Size of valid block (2^?)"; beam_block_bits "beam block size (2^?)"; y_beam_val "Y-beam value"; ant_bits "Number of antennas (2^?)"
  pow_sim_mux7 : Subsystem  mask{valid_block_bits=7+3, beam_block_bits=7, y_beam_val=6, ant_bits=3}
      mask prompts: valid_block_bits "Size of valid block (2^?)"; beam_block_bits "beam block size (2^?)"; y_beam_val "Y-beam value"; ant_bits "Number of antennas (2^?)"
  pow_sim_mux8 : Subsystem  mask{valid_block_bits=7+3, beam_block_bits=7, y_beam_val=7, ant_bits=3}
      mask prompts: valid_block_bits "Size of valid block (2^?)"; beam_block_bits "beam block size (2^?)"; y_beam_val "Y-beam value"; ant_bits "Number of antennas (2^?)"
  pow_sim_mux9 : Subsystem  mask{valid_block_bits=7+3, beam_block_bits=7, y_beam_val=8, ant_bits=3}
      mask prompts: valid_block_bits "Size of valid block (2^?)"; beam_block_bits "beam block size (2^?)"; y_beam_val "Y-beam value"; ant_bits "Number of antennas (2^?)"
  new_window_out : Outport
  dout0 : Outport  Port=2
  dout1 : Outport  Port=3
  dout2 : Outport  Port=4
  dout3 : Outport  Port=5
  dout4 : Outport  Port=6
  dout5 : Outport  Port=7
  dout6 : Outport  Port=8
  dout7 : Outport  Port=9
  dout8 : Outport  Port=10
  dout9 : Outport  Port=11
  dout10 : Outport  Port=12
  dout11 : Outport  Port=13
  dout12 : Outport  Port=14
  dout13 : Outport  Port=15
  dout14 : Outport  Port=16
  dout15 : Outport  Port=17
  wires:
  sync/1 -> [sync]
  new_window/1 -> [new_window], Delay/1
  [sync] -> pow_sim_mux1/1(sync)
  [new_window] -> pow_sim_mux1/4(new_window)
  tvg_en/1 -> [tvg_en]
  [tvg_en] -> pow_sim_mux1/3(tvg_en)
  din0/1 -> pow_sim_mux1/2(din)
  pow_sim_mux1/1(dout) -> dout0/1
  [sync] -> pow_sim_mux2/1(sync)
  [new_window] -> pow_sim_mux2/4(new_window)
  [tvg_en] -> pow_sim_mux2/3(tvg_en)
  din1/1 -> pow_sim_mux2/2(din)
  pow_sim_mux2/1(dout) -> dout1/1
  [sync] -> pow_sim_mux3/1(sync)
  [new_window] -> pow_sim_mux3/4(new_window)
  [tvg_en] -> pow_sim_mux3/3(tvg_en)
  din2/1 -> pow_sim_mux3/2(din)
  pow_sim_mux3/1(dout) -> dout2/1
  [sync] -> pow_sim_mux4/1(sync)
  [new_window] -> pow_sim_mux4/4(new_window)
  [tvg_en] -> pow_sim_mux4/3(tvg_en)
  din3/1 -> pow_sim_mux4/2(din)
  pow_sim_mux4/1(dout) -> dout3/1
  [sync] -> pow_sim_mux5/1(sync)
  [new_window] -> pow_sim_mux5/4(new_window)
  [tvg_en] -> pow_sim_mux5/3(tvg_en)
  din4/1 -> pow_sim_mux5/2(din)
  pow_sim_mux5/1(dout) -> dout4/1
  [sync] -> pow_sim_mux6/1(sync)
  [new_window] -> pow_sim_mux6/4(new_window)
  [tvg_en] -> pow_sim_mux6/3(tvg_en)
  din5/1 -> pow_sim_mux6/2(din)
  pow_sim_mux6/1(dout) -> dout5/1
  [sync] -> pow_sim_mux7/1(sync)
  [new_window] -> pow_sim_mux7/4(new_window)
  [tvg_en] -> pow_sim_mux7/3(tvg_en)
  din6/1 -> pow_sim_mux7/2(din)
  pow_sim_mux7/1(dout) -> dout6/1
  [sync] -> pow_sim_mux8/1(sync)
  [new_window] -> pow_sim_mux8/4(new_window)
  [tvg_en] -> pow_sim_mux8/3(tvg_en)
  din7/1 -> pow_sim_mux8/2(din)
  pow_sim_mux8/1(dout) -> dout7/1
  [sync] -> pow_sim_mux9/1(sync)
  [new_window] -> pow_sim_mux9/4(new_window)
  [tvg_en] -> pow_sim_mux9/3(tvg_en)
  din8/1 -> pow_sim_mux9/2(din)
  pow_sim_mux9/1(dout) -> dout8/1
  [sync] -> pow_sim_mux10/1(sync)
  [new_window] -> pow_sim_mux10/4(new_window)
  [tvg_en] -> pow_sim_mux10/3(tvg_en)
  din9/1 -> pow_sim_mux10/2(din)
  pow_sim_mux10/1(dout) -> dout9/1
  [sync] -> pow_sim_mux11/1(sync)
  [new_window] -> pow_sim_mux11/4(new_window)
  [tvg_en] -> pow_sim_mux11/3(tvg_en)
  din10/1 -> pow_sim_mux11/2(din)
  pow_sim_mux11/1(dout) -> dout10/1
  [sync] -> pow_sim_mux12/1(sync)
  [new_window] -> pow_sim_mux12/4(new_window)
  [tvg_en] -> pow_sim_mux12/3(tvg_en)
  din11/1 -> pow_sim_mux12/2(din)
  pow_sim_mux12/1(dout) -> dout11/1
  [sync] -> pow_sim_mux16/1(sync)
  [new_window] -> pow_sim_mux16/4(new_window)
  [tvg_en] -> pow_sim_mux16/3(tvg_en)
  din12/1 -> pow_sim_mux16/2(din)
  pow_sim_mux16/1(dout) -> dout12/1
  [sync] -> pow_sim_mux13/1(sync)
  [new_window] -> pow_sim_mux13/4(new_window)
  [tvg_en] -> pow_sim_mux13/3(tvg_en)
  din13/1 -> pow_sim_mux13/2(din)
  pow_sim_mux13/1(dout) -> dout13/1
  [sync] -> pow_sim_mux14/1(sync)
  [new_window] -> pow_sim_mux14/4(new_window)
  [tvg_en] -> pow_sim_mux14/3(tvg_en)
  din14/1 -> pow_sim_mux14/2(din)
  pow_sim_mux14/1(dout) -> dout14/1
  [sync] -> pow_sim_mux15/1(sync)
  [new_window] -> pow_sim_mux15/4(new_window)
  [tvg_en] -> pow_sim_mux15/3(tvg_en)
  din15/1 -> pow_sim_mux15/2(din)
  pow_sim_mux15/1(dout) -> dout15/1
  Delay/1 -> new_window_out/1

== system x64_32s_001_4ks/block_qdr_transpose ==
  note: Straight counter for
      | write address
  note: Alternating WR then RD
      | Only allow read on a sync cycle
  note: Reorder required as QDR performs
      | bursts of two 36-bit words
  note: delay: 2^(odepth+idepth+block_size) + 10(qdr_latency) + 1
      | (read command issued after write cycle)
  sync : Inport
  d : Inport  Port=2
  vld : Inport  Port=3
  Concat : xbsIndex_r4/Concat  num_inputs=4  dbl_ovrd=off  block_type=concat
      settings: block_version=10.1.3
  Constant : xbsIndex_r4/Constant  arith_type=Unsigned  const=15  n_bits=4  bin_pt=0  explicit_period=on  period=1  equ=P=C  opselect=C  inp2=PCIN>>17  opr=+  inp1=P  carry=CIN  dbl_ovrd=off  block_type=constant
  Delay : xbsIndex_r4/Delay  en=off  latency=10 + 1  dbl_ovrd=off  reg_retiming=on  block_type=delay
      settings: xl_area=[0, 0, 0, 0, 0, 0, 0]  block_version=9.2.01
  Inverter : xbsIndex_r4/Inverter  en=off  latency=0  dbl_ovrd=off  block_type=inv
  Inverter1 : xbsIndex_r4/Inverter  en=off  latency=0  dbl_ovrd=off  block_type=inv
  Logical : xbsIndex_r4/Logical  logical_function=AND  inputs=3  en=off  latency=0  precision=Full  arith_type=Unsigned  n_bits=16  bin_pt=0  align_bp=on  dbl_ovrd=off  block_type=logical
  Logical1 : xbsIndex_r4/Logical  logical_function=AND  inputs=2  en=off  latency=0  precision=Full  arith_type=Unsigned  n_bits=16  bin_pt=0  align_bp=on  dbl_ovrd=off  block_type=logical
  Logical2 : xbsIndex_r4/Logical  logical_function=AND  inputs=2  en=off  latency=0  precision=Full  arith_type=Unsigned  n_bits=16  bin_pt=0  align_bp=on  dbl_ovrd=off  block_type=logical
  Logical3 : xbsIndex_r4/Logical  logical_function=AND  inputs=2  en=off  latency=0  precision=Full  arith_type=Unsigned  n_bits=16  bin_pt=0  align_bp=on  dbl_ovrd=off  block_type=logical
  Mux : xbsIndex_r4/Mux  inputs=2  en=off  latency=0  precision=Full  arith_type=Unsigned  n_bits=16  bin_pt=14  quantization=Truncate  overflow=Wrap  dbl_ovrd=off  block_type=mux
  Slice : xbsIndex_r4/Slice  nbits=1  boolean_output=on  mode=Lower Bit Location + Width  bit1=0  base1=MSB of Input  bit0=0  base0=LSB of Input  dbl_ovrd=off  block_type=slice
      settings: infoedit="Extracts a given range of bits from each input sample and presents it at the output.  The output type is ordinarily unsigned with binary point at zero, but can be Boolean when the slice is one bit wide.<P><P>Hardware notes: In hardware this block costs nothing."  block_version=10.1.3
  Terminator{,1..3} (4 alike) : Terminator
  block_position : xbsIndex_r4/Slice  nbits=block_size-1  boolean_output=off  mode=Lower Bit Location + Width  bit1=9-1  base1=MSB of Input  bit0=0  base0=LSB of Input  dbl_ovrd=off  block_type=slice
      settings: block_version=10.1.3
  buffer_sel : xbsIndex_r4/Slice  nbits=1  boolean_output=off  mode=Upper Bit Location + Width  bit1=0  base1=MSB of Input  bit0=0  base0=LSB of Input  dbl_ovrd=off  block_type=slice
      settings: block_version=10.1.3
  chan_sel : xbsIndex_r4/Slice  nbits=idepth  boolean_output=off  mode=Lower Bit Location + Width  bit1=9-1  base1=MSB of Input  bit0=odepth+block_size-1  base0=LSB of Input  dbl_ovrd=off  block_type=slice
      settings: block_version=10.1.3
  ctrl_state : xbsIndex_r4/Register  init=0  rst=on  en=on  dbl_ovrd=off  block_type=register
  dump_sel : xbsIndex_r4/Slice  nbits=odepth  boolean_output=off  mode=Lower Bit Location + Width  bit1=0  base1=MSB of Input  bit0=block_size-1  base0=LSB of Input  dbl_ovrd=off  block_type=slice
      settings: block_version=10.1.3
  posedge : casper_library_misc/posedge  Tag=Rising Edge Detector  SystemSampleTime=-1
  qdr : xps_library/qdr  Tag=xps:qdr  SystemSampleTime=-1  which_qdr=qdr1  qdr_awidth=min(odepth+idepth+block_size+1,14)  use_sniffer=off
  rd_addr : xbsIndex_r4/Counter  cnt_type=Free Running  cnt_to=Inf  operation=Up  start_count=2^(odepth + idepth + block_size - 1)  cnt_by_val=1  arith_type=Unsigned  n_bits=(idepth + odepth + block_size + 1) - 1  bin_pt=0  load_pin=off  rst=on  en=on  explicit_period=on  period=1  dbl_ovrd=off  use_behavioral_HDL=off  implementation=Fabric  use_rpm=off  block_type=counter
  sync_delay : casper_library_delays/sync_delay  SystemSampleTime=-1  DelayLen=2^(idepth + odepth + block_size) + 10 + 1
  wr_addr : xbsIndex_r4/Counter  cnt_type=Free Running  cnt_to=Inf  operation=Up  start_count=0  cnt_by_val=1  arith_type=Unsigned  n_bits=(idepth + odepth + block_size + 1) - 1  bin_pt=0  load_pin=off  rst=on  en=on  explicit_period=on  period=1  dbl_ovrd=off  use_behavioral_HDL=off  implementation=Fabric  use_rpm=off  block_type=counter
  sync_out : Outport
  q : Outport  Port=2
  vld_out : Outport  Port=3
  wires:
  [vld] -> Delay/1
  Delay/1 -> vld_out/1
  [vld] -> ctrl_state/3
  [vld] -> Logical2/2
  [WR] -> Logical2/1
  Logical2/1 -> wr_addr/2
  [vld] -> Logical1/2
  [RD] -> Logical1/1
  Logical1/1 -> rd_addr/2
  vld/1 -> [vld]
  block_position/1 -> Concat/4
  qdr/1 -> q/1
  sync_delay/1 -> sync_out/1
  chan_sel/1 -> Concat/3
  dump_sel/1 -> Concat/2
  rd_addr/1 -> block_position/1, dump_sel/1, buffer_sel/1, chan_sel/1
  buffer_sel/1 -> Concat/1
  Concat/1 -> Mux/3
  Logical/1 -> [WR]
  Inverter1/1 -> Logical/2
  posedge/1 -> [synci]
  [synci] -> Inverter1/1
  Slice/1 -> Inverter/1, Logical3/1
  [synci] -> sync_delay/1
  qdr/2 -> Terminator3/1
  qdr/3 -> Terminator2/1
  qdr/4 -> Terminator1/1
  qdr/5 -> Terminator/1
  d/1 -> qdr/5
  Mux/1 -> qdr/4
  Constant/1 -> qdr/3
  [WR] -> qdr/2
  [RD] -> qdr/1
  wr_addr/1 -> Mux/2
  [RD] -> Mux/1
  Inverter/1 -> Logical/1, ctrl_state/1
  [synci] -> rd_addr/1
  [synci] -> wr_addr/1
  [synci] -> ctrl_state/2
  sync/1 -> posedge/1
  ctrl_state/1 -> Slice/1
  Logical3/1 -> [RD]
  [vld] -> Logical3/2, Logical/3
